simulink model "model"  (R2020b)
settings: Solver=FixedStepAuto  StartTime=0.0  StopTime=10  FixedStep=0.01  RelTol=1e-3
settings Model: Creator=Arvind Hishikar  LastModifiedBy=Arvind Hishikar

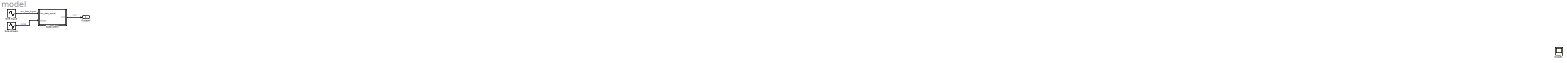
== system model ==
  Sine Wave : Sin  Amplitude=15  Frequency=100  SampleTime=0
  Sine Wave1 : Sin  Amplitude=3  Frequency=62356  SampleTime=0
  Subsystem : Subsystem
  Outport : Outport
  Scope1 : Scope
  wires:
  Sine Wave/1 -> Subsystem/1(low_freq_signal)  "low_freq_signal"
  Sine Wave1/1 -> Subsystem/2(noise)  "noise"
  Subsystem/1(y(n)) -> Outport/1  "y(n)"

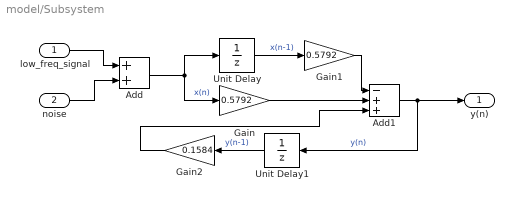
== system model/Subsystem ==
  low_freq_signal : Inport
  noise : Inport  Port=2
  Add : Sum
  Add1 : Sum  Inputs=-++
  Gain : Gain  Gain=0.5792
  Gain1 : Gain  Gain=0.5792
  Gain2 : Gain  Gain=0.1584
  Unit Delay : UnitDelay  SampleTime=-1  HasFrameUpgradeWarning=on
  Unit Delay1 : UnitDelay  SampleTime=-1  HasFrameUpgradeWarning=on
  y(n) : Outport
  wires:
  Gain2/1 -> Add1/3
  Add1/1 -> y(n)/1, Unit Delay1/1  "y(n)"
  Unit Delay/1 -> Gain1/1  "x(n-1)"
  Gain1/1 -> Add1/1
  Gain/1 -> Add1/2
  low_freq_signal/1 -> Add/1
  Add/1 -> Gain/1, Unit Delay/1  "x(n)"
  Unit Delay1/1 -> Gain2/1  "y(n-1)"
  noise/1 -> Add/2
